simulink model "CCMMotor"  (R2022b) (continued)
== system CCMMotor/CCMMotor_Runnable_sys == (continued)
      settings: EnsureOutportIsVirtual=on
  AirFlapPosnFrntActForRowFirstForActrVentLe_AirFlapPosnFrntActForRowFirstForActrVentLe_write : Outport  Port=35
      settings: EnsureOutportIsVirtual=on
  AirFlapPosnFrntActForRowFirstForActrVentRi_AirFlapPosnFrntActForRowFirstForActrVentRi_write : Outport  Port=36
      settings: EnsureOutportIsVirtual=on
  AirFlapPosnFrntActForRowFirstForActrVentSideLe_AirFlapPosnFrntActForRowFirstForActrVentSideLe_write : Outport  Port=37
      settings: EnsureOutportIsVirtual=on
  AirFlapPosnFrntActForRowFirstForActrVentSideRi_AirFlapPosnFrntActForRowFirstForActrVentSideRi_write : Outport  Port=38
      settings: EnsureOutportIsVirtual=on
  AirFlapPosnFrntActForRowFirstForOsa_AirFlapPosnFrntActForRowFirstForOsa_write : Outport  Port=39
      settings: EnsureOutportIsVirtual=on
  AirFlapPosnFrntActForRowFirstForRecirc_AirFlapPosnFrntActForRowFirstForRecirc_write : Outport  Port=40
      settings: EnsureOutportIsVirtual=on
  AirFlapPosnFrntActForRowSecForActrTLe_AirFlapPosnFrntActForRowSecForActrTLe_write : Outport  Port=41
      settings: EnsureOutportIsVirtual=on
  AirFlapPosnFrntActForRowSecForActrTRi_AirFlapPosnFrntActForRowSecForActrTRi_write : Outport  Port=42
      settings: EnsureOutportIsVirtual=on
  CcmElecAirDirCrtlReqDrvrLePosX_CcmElecAirDirCrtlReqDrvrLePosX_write : Outport  Port=43
      settings: EnsureOutportIsVirtual=on
  CcmElecAirDirCrtlReqDrvrLePosY_CcmElecAirDirCrtlReqDrvrLePosY_write : Outport  Port=44
      settings: EnsureOutportIsVirtual=on
  CcmElecAirDirCrtlReqDrvrRiPosX_CcmElecAirDirCrtlReqDrvrRiPosX_write : Outport  Port=45
      settings: EnsureOutportIsVirtual=on
  CcmElecAirDirCrtlReqDrvrRiPosY_CcmElecAirDirCrtlReqDrvrRiPosY_write : Outport  Port=46
      settings: EnsureOutportIsVirtual=on
  CcmElecAirDirCrtlReqPassLePosX_CcmElecAirDirCrtlReqPassLePosX_write : Outport  Port=47
      settings: EnsureOutportIsVirtual=on
  CcmElecAirDirCrtlReqPassLePosY_CcmElecAirDirCrtlReqPassLePosY_write : Outport  Port=48
      settings: EnsureOutportIsVirtual=on
  CcmElecAirDirCrtlReqPassRiPosX_CcmElecAirDirCrtlReqPassRiPosX_write : Outport  Port=49
      settings: EnsureOutportIsVirtual=on
  CcmElecAirDirCrtlReqPassRiPosY_CcmElecAirDirCrtlReqPassRiPosY_write : Outport  Port=50
      settings: EnsureOutportIsVirtual=on
  FrntVGAerror_FrntVGAerror_write : Outport  Port=51
      settings: EnsureOutportIsVirtual=on
  RearVGAerror_RearVGAerror_write : Outport  Port=52
      settings: EnsureOutportIsVirtual=on
  VentnDrvrLeXStsRsp_VentnDrvrLeXStsRsp_write : Outport  Port=53
      settings: EnsureOutportIsVirtual=on
  VentnDrvrLeYStsRsp_VentnDrvrLeYStsRsp_write : Outport  Port=54
      settings: EnsureOutportIsVirtual=on
  VentnDrvrRiXStsRsp_VentnDrvrRiXStsRsp_write : Outport  Port=55
      settings: EnsureOutportIsVirtual=on
  VentnDrvrRiYStsRsp_VentnDrvrRiYStsRsp_write : Outport  Port=56
      settings: EnsureOutportIsVirtual=on
  VentnPassLeXStsRsp_VentnPassLeXStsRsp_write : Outport  Port=57
      settings: EnsureOutportIsVirtual=on
  VentnPassLeYStsRsp_VentnPassLeYStsRsp_write : Outport  Port=58
      settings: EnsureOutportIsVirtual=on
  VentnPassRiXStsRsp_VentnPassRiXStsRsp_write : Outport  Port=59
      settings: EnsureOutportIsVirtual=on
  VentnPassRiYStsRsp_VentnPassRiYStsRsp_write : Outport  Port=60
      settings: EnsureOutportIsVirtual=on
  VentnSecLeXStsRsp_VentnSecLeXStsRsp_write : Outport  Port=61
      settings: EnsureOutportIsVirtual=on
  VentnSecLeYStsRsp_VentnSecLeYStsRsp_write : Outport  Port=62
      settings: EnsureOutportIsVirtual=on
  VentnSecRiXStsRsp_VentnSecRiXStsRsp_write : Outport  Port=63
      settings: EnsureOutportIsVirtual=on
  VentnSecRiYStsRsp_VentnSecRiYStsRsp_write : Outport  Port=64
      settings: EnsureOutportIsVirtual=on
  wires:
  ActrFrntReqForRowSecModDistbnRi_ActrFrntReqForRowSecModDistbnRi_read/1 -> Terminator_1/1
  AirFlapPosnFrntReqForRowFirstForActrFlrLe_AirFlapPosnFrntReqForRowFirstForActrFlrLe_read/1 -> Terminator_2/1
  AirFlapPosnFrntReqForRowFirstForActrFlrRi_AirFlapPosnFrntReqForRowFirstForActrFlrRi_read/1 -> Terminator_3/1
  AirFlapPosnFrntReqForRowFirstForActrVentLe_AirFlapPosnFrntReqForRowFirstForActrVentLe_read/1 -> Terminator_4/1
  AirFlapPosnFrntReqForRowFirstForActrVentRi_AirFlapPosnFrntReqForRowFirstForActrVentRi_read/1 -> Terminator_5/1
  AirFlapPosnFrntReqForRowFirstForActrVentSideLe_AirFlapPosnFrntReqForRowFirstForActrVentSideLe_read/1 -> Terminator_6/1
  AirFlapPosnFrntReqForRowFirstForActrVentSideRi_AirFlapPosnFrntReqForRowFirstForActrVentSideRi_read/1 -> Terminator_7/1
  AirFlapPosnFrntReqForRowFirstForOsa_AirFlapPosnFrntReqForRowFirstForOsa_read/1 -> Terminator_8/1
  AirFlapPosnFrntReqForRowFirstForRecirc_AirFlapPosnFrntReqForRowFirstForRecirc_read/1 -> Terminator_9/1
  AirFlapPosnFrntReqForRowSecForActrTRi_AirFlapPosnFrntReqForRowSecForActrTRi_read/1 -> Terminator_10/1
  PwrMdAllMtrSlp_PwrMdAllMtrSlp_read/1 -> Terminator_11/1
  SysServCarCfgSts_SysServCarID_read/1 -> Terminator_12/1
  SysServCarCfgSts_SysServCCPAQSSts_read/1 -> Terminator_13/1
  SysServCarCfgSts_SysServCCPHEXSensorSts_read/1 -> Terminator_14/1
  SysServCarCfgSts_SysServCCPLin2AFUSts_read/1 -> Terminator_15/1
  SysServCarCfgSts_SysServCCPLin2AIUSts_read/1 -> Terminator_16/1
  SysServCarCfgSts_SysServCCPLin2CDSSts_read/1 -> Terminator_17/1
  SysServCarCfgSts_SysServCCPLin2PMSISts_read/1 -> Terminator_18/1
  SysServCarCfgSts_SysServCCPLin3VGASts_read/1 -> Terminator_19/1
  SysServCarCfgSts_SysServDUAirVentSnrSts_read/1 -> Terminator_20/1
  SysServCarCfgSts_SysServDUBlowerPwmSignSts_read/1 -> Terminator_21/1
  SysServCarCfgSts_SysServDUBlowerPwmSts_read/1 -> Terminator_22/1
  SysServCarCfgSts_SysServDUDCDefrostSts_read/1 -> Terminator_23/1
  SysServCarCfgSts_SysServDUDCModeSts_read/1 -> Terminator_24/1
  SysServCarCfgSts_SysServDUDCRecSts_read/1 -> Terminator_25/1
  SysServCarCfgSts_SysServDUDCSModeSts_read/1 -> Terminator_26/1
  SysServCarCfgSts_SysServDUDCSTLSts_read/1 -> Terminator_27/1
  SysServCarCfgSts_SysServDUDCTLSts_read/1 -> Terminator_28/1
  SysServCarCfgSts_SysServDUDCTRSts_read/1 -> Terminator_29/1
  SysServCarCfgSts_SysServDUFLFootSts_read/1 -> Terminator_30/1
  SysServCarCfgSts_SysServDUFLVentSts_read/1 -> Terminator_31/1
  SysServCarCfgSts_SysServDUFRFootSts_read/1 -> Terminator_32/1
  SysServCarCfgSts_SysServDUFRVentSts_read/1 -> Terminator_33/1
  SysServCarCfgSts_SysServDUIncarMotorSts_read/1 -> Terminator_34/1
  SysServCarCfgSts_SysServDULin1HBMFSts_read/1 -> Terminator_35/1
  SysServCarCfgSts_SysServDULin4HFAFSts_read/1 -> Terminator_36/1
  SysServCarCfgSts_SysServDUSFLFootSts_read/1 -> Terminator_37/1
  SysServCarCfgSts_SysServDUSFLVentSts_read/1 -> Terminator_38/1
  SysServCarCfgSts_SysServDUSFRFootSts_read/1 -> Terminator_39/1
  SysServCarCfgSts_SysServDUSFRVentSts_read/1 -> Terminator_40/1
  SysServCarCfgSts_SysServDUSnsrForFCmptmtTSts_read/1 -> Terminator_41/1
  SysServCarCfgSts_SysServDUSnsrForRair_read/1 -> Terminator_42/1
  SysServCarCfgSts_SysServDUSnsrForRCmptmtTSts_read/1 -> Terminator_43/1
  VehModMngtGlbSafe1_VehModMngtGlbSafe1_read/1 -> Terminator_44/1
  ReqForActrCal_ReqForActrCal_read/1 -> Terminator_45/1
  ActrFrntReqForRowFirstLeModDistbn_ActrFrntReqForRowFirstLeModDistbn_read/1 -> Terminator_46/1
  ActrFrntReqForRowFirstLeModDistbn_ActrFrntReqForRowFirstLeModDistbn_IsUpdated_read/1 -> Terminator_47/1
  ActrFrntReqForRowFirstModDistbn_ActrFrntReqForRowFirstModDistbn_read/1 -> Terminator_48/1
  ActrFrntReqForRowFirstModDistbn_ActrFrntReqForRowFirstModDistbn_IsUpdated_read/1 -> Terminator_49/1
  ActrFrntReqForRowSecModDistbnLe_ActrFrntReqForRowSecModDistbnLe_read/1 -> Terminator_50/1
  ActrFrntReqForRowSecModDistbnLe_ActrFrntReqForRowSecModDistbnLe_IsUpdated_read/1 -> Terminator_51/1
  ActrPosnFrnReqForOsaAndRecirc_ActrPosnFrnReqForOsaAndRecirc_read/1 -> Terminator_52/1
  ActrPosnFrnReqForOsaAndRecirc_ActrPosnFrnReqForOsaAndRecirc_IsUpdated_read/1 -> Terminator_53/1
  ActrReqForModDistbnRe_ActrReqForModDistbnRe_read/1 -> Terminator_54/1
  ActrReqForModDistbnRe_ActrReqForModDistbnRe_IsUpdated_read/1 -> Terminator_55/1
  AirFlapPosnFrntReqForRowFirstForActrDefrst_AirFlapPosnFrntReqForRowFirstForActrDefrst_read/1 -> Terminator_56/1
  AirFlapPosnFrntReqForRowFirstForActrDefrst_AirFlapPosnFrntReqForRowFirstForActrDefrst_IsUpdated_read/1 -> Terminator_57/1
  AirFlapPosnFrntReqForRowFirstForActrTLe_AirFlapPosnFrntReqForRowFirstForActrTLe_read/1 -> Terminator_58/1
  AirFlapPosnFrntReqForRowFirstForActrTLe_AirFlapPosnFrntReqForRowFirstForActrTLe_IsUpdated_read/1 -> Terminator_59/1
  AirFlapPosnFrntReqForRowFirstForActrTRi_AirFlapPosnFrntReqForRowFirstForActrTRi_read/1 -> Terminator_60/1
  AirFlapPosnFrntReqForRowFirstForActrTRi_AirFlapPosnFrntReqForRowFirstForActrTRi_IsUpdated_read/1 -> Terminator_61/1
  AirFlapPosnFrntReqForRowSecForActrTLe_AirFlapPosnFrntReqForRowSecForActrTLe_read/1 -> Terminator_62/1
  AirFlapPosnFrntReqForRowSecForActrTLe_AirFlapPosnFrntReqForRowSecForActrTLe_IsUpdated_read/1 -> Terminator_63/1
  CarCfgForClimaCtrl_CarCfgForAirClng_read/1 -> Terminator_64/1
  CarCfgForClimaCtrl_CarCfgForAirClnrAdv_read/1 -> Terminator_65/1
  CarCfgForClimaCtrl_CarCfgForAirFrag_read/1 -> Terminator_66/1
  CarCfgForClimaCtrl_CarCfgForAirMtrlSnsr_read/1 -> Terminator_67/1
  CarCfgForClimaCtrl_CarCfgForAirQly_read/1 -> Terminator_68/1
  CarCfgForClimaCtrl_CarCfgForAmntOfSeat_read/1 -> Terminator_69/1
  CarCfgForClimaCtrl_CarCfgForAutDemst_read/1 -> Terminator_70/1
  CarCfgForClimaCtrl_CarCfgForAuxWtrPmp_read/1 -> Terminator_71/1
  CarCfgForClimaCtrl_CarCfgForBRAND_read/1 -> Terminator_72/1
  CarCfgForClimaCtrl_CarCfgForBypVlvInHvac_read/1 -> Terminator_73/1
  CarCfgForClimaCtrl_CarCfgForClsVlvInHvac_read/1 -> Terminator_74/1
  CarCfgForClimaCtrl_CarCfgForCmprAcTyp_read/1 -> Terminator_75/1
  CarCfgForClimaCtrl_CarCfgForCmptmtClng_read/1 -> Terminator_76/1
  CarCfgForClimaCtrl_CarCfgForElectricLiftingModule_read/1 -> Terminator_77/1
  CarCfgForClimaCtrl_CarCfgForElecVentAdjmt_read/1 -> Terminator_78/1
  CarCfgForClimaCtrl_CarCfgForExtVentnClimaCtrl_read/1 -> Terminator_79/1
  CarCfgForClimaCtrl_CarCfgForFlcTyp_read/1 -> Terminator_80/1
  CarCfgForClimaCtrl_CarCfgForFoldCSD_read/1 -> Terminator_81/1
  CarCfgForClimaCtrl_CarCfgForFuTyp_read/1 -> Terminator_82/1
  CarCfgForClimaCtrl_CarCfgForGlassTyp_read/1 -> Terminator_83/1
  CarCfgForClimaCtrl_CarCfgForHeadRadar_read/1 -> Terminator_84/1
  CarCfgForClimaCtrl_CarCfgForHeatPmp_read/1 -> Terminator_85/1
  CarCfgForClimaCtrl_CarCfgForHeatrDurgDrvg_read/1 -> Terminator_86/1
  CarCfgForClimaCtrl_CarCfgForHeatrTyp_read/1 -> Terminator_87/1
  CarCfgForClimaCtrl_CarCfgForHvacFilTyp_read/1 -> Terminator_88/1
  CarCfgForClimaCtrl_CarCfgForHvacFlrSnsrForT_read/1 -> Terminator_89/1
  CarCfgForClimaCtrl_CarCfgForHvacHeatrSnsrT_read/1 -> Terminator_90/1
  CarCfgForClimaCtrl_CarCfgForHvacRe_read/1 -> Terminator_91/1
  CarCfgForClimaCtrl_CarCfgForHvacTyp_read/1 -> Terminator_92/1
  CarCfgForClimaCtrl_CarCfgForInteriorViewCamera_read/1 -> Terminator_93/1
  CarCfgForClimaCtrl_CarCfgForLifeDetection_read/1 -> Terminator_94/1
  CarCfgForClimaCtrl_CarCfgForLoVoltElecCfg_read/1 -> Terminator_95/1
  CarCfgForClimaCtrl_CarCfgForNavSysandIntnmap_read/1 -> Terminator_96/1
  CarCfgForClimaCtrl_CarCfgForNrOfDoor_read/1 -> Terminator_97/1
  CarCfgForClimaCtrl_CarCfgForPipeInFuTank_read/1 -> Terminator_98/1
  CarCfgForClimaCtrl_CarCfgForPowerSeats_read/1 -> Terminator_99/1
  CarCfgForClimaCtrl_CarCfgForPreClng_read/1 -> Terminator_100/1
  CarCfgForClimaCtrl_CarCfgForPrkgCDSNotAvl_read/1 -> Terminator_101/1
  CarCfgForClimaCtrl_CarCfgForPrkgClima_read/1 -> Terminator_102/1
  CarCfgForClimaCtrl_CarCfgForPrpsnTyp_read/1 -> Terminator_103/1
  CarCfgForClimaCtrl_CarCfgForPTCInFrontHVAC_read/1 -> Terminator_104/1
  CarCfgForClimaCtrl_CarCfgForRemEngStrtPreCdn_read/1 -> Terminator_105/1
  CarCfgForClimaCtrl_CarCfgForRoofHatch_read/1 -> Terminator_106/1
  CarCfgForClimaCtrl_CarCfgForSeatHeatgCal_read/1 -> Terminator_107/1
  CarCfgForClimaCtrl_CarCfgForSeatHeatgForRowFirst_read/1 -> Terminator_108/1
  CarCfgForClimaCtrl_CarCfgForSeatHeatgForRowSec_read/1 -> Terminator_109/1
  CarCfgForClimaCtrl_CarCfgForSeatVentnForRowFirst_read/1 -> Terminator_110/1
  CarCfgForClimaCtrl_CarCfgForSeatVentnForRowSec_read/1 -> Terminator_111/1
  CarCfgForClimaCtrl_CarCfgForSecRowVentnCenter_read/1 -> Terminator_112/1
  CarCfgForClimaCtrl_CarCfgForSecRowVentnSide_read/1 -> Terminator_113/1
  CarCfgForClimaCtrl_CarCfgForSmallFlowCyc_read/1 -> Terminator_114/1
  CarCfgForClimaCtrl_CarCfgForSmkSnsr_read/1 -> Terminator_115/1
  CarCfgForClimaCtrl_CarCfgForSnsrForCmptmtT_read/1 -> Terminator_116/1
  CarCfgForClimaCtrl_CarCfgForSnsrHumPrsnt_read/1 -> Terminator_117/1
  CarCfgForClimaCtrl_CarCfgForStartDevice_read/1 -> Terminator_118/1
  CarCfgForClimaCtrl_CarCfgForSteerWhlHeatg_read/1 -> Terminator_119/1
  CarCfgForClimaCtrl_CarCfgForSteerWhlPosn_read/1 -> Terminator_120/1
  CarCfgForClimaCtrl_CarCfgForSunSnsr_read/1 -> Terminator_121/1
  CarCfgForClimaCtrl_CarCfgForTelmNodTyp_read/1 -> Terminator_122/1
  CarCfgForClimaCtrl_CarCfgForTEMPEREDSTORAGEBOX_read/1 -> Terminator_123/1
  CarCfgForClimaCtrl_CarCfgForThermMngt_read/1 -> Terminator_124/1
  CarCfgForClimaCtrl_CarCfgForVehTyp_read/1 -> Terminator_125/1
  CarCfgForClimaCtrl_CarCfgForVentLiftMotorExiSts_read/1 -> Terminator_126/1
  CarCfgForClimaCtrl_CarCfgForVgaMotorExistSts_read/1 -> Terminator_127/1
  CarCfgForClimaCtrl_CarCfgForWadingAssistSystem_read/1 -> Terminator_128/1
  CarCfgForClimaCtrl_CarCfgForWindHeatd_read/1 -> Terminator_129/1
  CarCfgForClimaCtrl_CarCfgWindFollowHumanMov_read/1 -> Terminator_130/1
  SysServCarCfgSts_SysServCCPBGMNum_read/1 -> Terminator_131/1
  SysServCarCfgSts_SysServCCPLin2AQSSts_read/1 -> Terminator_132/1
  SysServCarCfgSts_SysServDUBlowerPwmSignTypeSts_read/1 -> Terminator_133/1
  SysServCarCfgSts_SysServDUDCFLMdeSts_read/1 -> Terminator_134/1
  SysServCarCfgSts_SysServDULin4HFAFStsH_read/1 -> Terminator_135/1
  SysServCarCfgSts_SysServDUReBlowerPwmSignSts_read/1 -> Terminator_136/1
  SysServCarCfgSts_SysServDUReBlowerPwmSts_read/1 -> Terminator_137/1
  SysServCarCfgSts_SysServFrntHEXSnsrPosn_read/1 -> Terminator_138/1
  SysServCarCfgSts_SysServLowSideRETXVSts_read/1 -> Terminator_139/1
  SysServCarCfgSts_SysServReHEXSnsrPosn_read/1 -> Terminator_140/1
  SysServCarCfgSts_SysServRHFASts_read/1 -> Terminator_141/1
  SysServCarCfgSts_SysServSnsrForFCmptmtTType_read/1 -> Terminator_142/1
  VentLiftModCaliReq_VentLiftModCaliReq_read/1 -> Terminator_143/1
  VentnDrvrLeXPsonReq_VentnDrvrLeXPsonReq_read/1 -> Terminator_144/1
  VentnDrvrLeXPsonReq_VentnDrvrLeXPsonReq_IsUpdated_read/1 -> Terminator_145/1
  VentnDrvrLeYPsonReq_VentnDrvrLeYPsonReq_read/1 -> Terminator_146/1
  VentnDrvrLeYPsonReq_VentnDrvrLeYPsonReq_IsUpdated_read/1 -> Terminator_147/1
  VentnDrvrRiXPsonReq_VentnDrvrRiXPsonReq_read/1 -> Terminator_148/1
  VentnDrvrRiXPsonReq_VentnDrvrRiXPsonReq_IsUpdated_read/1 -> Terminator_149/1
  VentnDrvrRiYPsonReq_VentnDrvrRiYPsonReq_read/1 -> Terminator_150/1
  VentnDrvrRiYPsonReq_VentnDrvrRiYPsonReq_IsUpdated_read/1 -> Terminator_151/1
  VentnLiftModPosnReq_VentnLiftModPosnReq_read/1 -> Terminator_152/1
  VentnLiftModPosnReq_VentnLiftModPosnReq_IsUpdated_read/1 -> Terminator_153/1
  VentnPassLeXPsonReq_VentnPassLeXPsonReq_read/1 -> Terminator_154/1
  VentnPassLeXPsonReq_VentnPassLeXPsonReq_IsUpdated_read/1 -> Terminator_155/1
  VentnPassLeYPsonReq_VentnPassLeYPsonReq_read/1 -> Terminator_156/1
  VentnPassLeYPsonReq_VentnPassLeYPsonReq_IsUpdated_read/1 -> Terminator_157/1
  VentnPassRiXPsonReq_VentnPassRiXPsonReq_read/1 -> Terminator_158/1
  VentnPassRiXPsonReq_VentnPassRiXPsonReq_IsUpdated_read/1 -> Terminator_159/1
  VentnPassRiYPsonReq_VentnPassRiYPsonReq_read/1 -> Terminator_160/1
  VentnPassRiYPsonReq_VentnPassRiYPsonReq_IsUpdated_read/1 -> Terminator_161/1
  VentnSecLeXPosnReq_VentnSecLeXPosnReq_read/1 -> Terminator_162/1
  VentnSecLeXPosnReq_VentnSecLeXPosnReq_IsUpdated_read/1 -> Terminator_163/1
  VentnSecLeYPosnReq_VentnSecLeYPosnReq_read/1 -> Terminator_164/1
  VentnSecLeYPosnReq_VentnSecLeYPosnReq_IsUpdated_read/1 -> Terminator_165/1
  VentnSecRiXPosnReq_VentnSecRiXPosnReq_read/1 -> Terminator_166/1
  VentnSecRiXPosnReq_VentnSecRiXPosnReq_IsUpdated_read/1 -> Terminator_167/1
  VentnSecRiYPosnReq_VentnSecRiYPosnReq_read/1 -> Terminator_168/1
  VentnSecRiYPosnReq_VentnSecRiYPosnReq_IsUpdated_read/1 -> Terminator_169/1
  WtrBafePsonReq_WtrBafePsonReq_read/1 -> Terminator_170/1
  BswLifeCycle_LifeCycle_read/1 -> Terminator_171/1
  DcMotorParaService_DcMotorAllowSleepSts/1 -> Terminator_172/1
  DcMotorParaService_DcMotorAllowSleepSts/2 -> Terminator_173/1
  Ground_1/1 -> DcMotorParaService_GetMotorActualPositon/1
  DcMotorParaService_GetMotorActualPositon/1 -> Terminator_174/1
  DcMotorParaService_GetMotorActualPositon/2 -> Terminator_175/1
  DcMotorParaService_GetMotorCailLearnSts/1 -> Terminator_176/1
  DcMotorParaService_GetMotorCailLearnSts/2 -> Terminator_177/1
  Ground_2/1 -> DcMotorParaService_GetMotorCalibrationStatus/1
  DcMotorParaService_GetMotorCalibrationStatus/1 -> Terminator_178/1
  DcMotorParaService_GetMotorCalibrationStatus/2 -> Terminator_179/1
  Ground_3/1 -> DcMotorParaService_GetMotorDidWorkingStatus/1
  DcMotorParaService_GetMotorDidWorkingStatus/1 -> Terminator_180/1
  DcMotorParaService_GetMotorDidWorkingStatus/2 -> Terminator_181/1
  Ground_4/1 -> DcMotorParaService_GetMotorEndPosition/1
  DcMotorParaService_GetMotorEndPosition/1 -> Terminator_182/1
  DcMotorParaService_GetMotorEndPosition/2 -> Terminator_183/1
  Ground_5/1 -> DcMotorParaService_GetMotorRequestPercent/1
  DcMotorParaService_GetMotorRequestPercent/1 -> Terminator_184/1
  DcMotorParaService_GetMotorRequestPercent/2 -> Terminator_185/1
  DcMotorParaService_GetMotorRoutineCailSts/1 -> Terminator_186/1
  DcMotorParaService_GetMotorRoutineCailSts/2 -> Terminator_187/1
  Ground_6/1 -> DcMotorParaService_GetMotorRunTime/1
  DcMotorParaService_GetMotorRunTime/1 -> Terminator_188/1
  DcMotorParaService_GetMotorRunTime/2 -> Terminator_189/1
  Ground_7/1 -> DcMotorParaService_GetMotorWorkingStatus/1
  DcMotorParaService_GetMotorWorkingStatus/1 -> Terminator_190/1
  DcMotorParaService_GetMotorWorkingStatus/2 -> Terminator_191/1
  Ground_8/1 -> DcMotorParaService_GetMotorZeroPosition/1
  DcMotorParaService_GetMotorZeroPosition/1 -> Terminator_192/1
  DcMotorParaService_GetMotorZeroPosition/2 -> Terminator_193/1
  DcMotorParaService_GetRamAirErrorStatus/1 -> Terminator_194/1
  DcMotorParaService_GetRamAirErrorStatus/2 -> Terminator_195/1
  Ground_9/1 -> DcMotorParaService_SetMotorEndPosition/1
  Ground_10/1 -> DcMotorParaService_SetMotorEndPosition/2
  DcMotorParaService_SetMotorEndPosition/1 -> Terminator_196/1
  Ground_11/1 -> DcMotorParaService_SetMotorRequestPercent/1
  Ground_12/1 -> DcMotorParaService_SetMotorRequestPercent/2
  DcMotorParaService_SetMotorRequestPercent/1 -> Terminator_197/1
  DcMotorParaService_SetMotorRoutineCail/1 -> Terminator_198/1
  Ground_13/1 -> DcMotorParaService_SetMotorRoutineCailSts/1
  DcMotorParaService_SetMotorRoutineCailSts/1 -> Terminator_199/1
  Ground_14/1 -> DcMotorParaService_SetMotorRunTime/1
  Ground_15/1 -> DcMotorParaService_SetMotorRunTime/2
  DcMotorParaService_SetMotorRunTime/1 -> Terminator_200/1
  Ground_16/1 -> DcMotorParaService_SetMotorZeroPosition/1
  Ground_17/1 -> DcMotorParaService_SetMotorZeroPosition/2
  DcMotorParaService_SetMotorZeroPosition/1 -> Terminator_201/1
  DcMtrCaliServive_SetAllCalibrate/1 -> Terminator_202/1
  Ground_18/1 -> DcMtrCaliServive_SetMotorCalibrate/1
  DcMtrCaliServive_SetMotorCalibrate/1 -> Terminator_203/1
  HfaCaliService_SetAllCalibrate/1 -> Terminator_204/1
  HfaCaliService_SetAllParaInit/1 -> Terminator_205/1
  Ground_19/1 -> HfaCaliService_SetMotorCalibrate/1
  HfaCaliService_SetMotorCalibrate/1 -> Terminator_206/1
  Ground_20/1 -> HfaCaliService_SetMotorParaInit/1
  HfaCaliService_SetMotorParaInit/1 -> Terminator_207/1
  HfaParaService_GetAllMotorCaliStatus/1 -> Terminator_208/1
  HfaParaService_GetAllMotorCaliStatus/2 -> Terminator_209/1
  HfaParaService_GetCailRoutineStatus/1 -> Terminator_210/1
  HfaParaService_GetCailRoutineStatus/2 -> Terminator_211/1
  HfaParaService_GetFreshRecErrorStatus/1 -> Terminator_212/1
  HfaParaService_GetFreshRecErrorStatus/2 -> Terminator_213/1
  HfaParaService_GetMotorAllowSleepSts/1 -> Terminator_214/1
  HfaParaService_GetMotorAllowSleepSts/2 -> Terminator_215/1
  Ground_21/1 -> HfaParaService_GetMotorCaliStatus/1
  HfaParaService_GetMotorCaliStatus/1 -> Terminator_216/1
  HfaParaService_GetMotorCaliStatus/2 -> Terminator_217/1
  Ground_22/1 -> HfaParaService_GetMotorCurrentPosition/1
  HfaParaService_GetMotorCurrentPosition/1 -> Terminator_218/1
  HfaParaService_GetMotorCurrentPosition/2 -> Terminator_219/1
  Ground_23/1 -> HfaParaService_GetMotorDidWorkingStatus/1
  HfaParaService_GetMotorDidWorkingStatus/1 -> Terminator_220/1
  HfaParaService_GetMotorDidWorkingStatus/2 -> Terminator_221/1
  Ground_24/1 -> HfaParaService_GetMotorMoveCnt/1
  HfaParaService_GetMotorMoveCnt/1 -> Terminator_222/1
  HfaParaService_GetMotorMoveCnt/2 -> Terminator_223/1
  Ground_25/1 -> HfaParaService_GetMotorReqPosition/1
  HfaParaService_GetMotorReqPosition/1 -> Terminator_224/1
  HfaParaService_GetMotorReqPosition/2 -> Terminator_225/1
  Ground_26/1 -> HfaParaService_GetMotorStepRange/1
  HfaParaService_GetMotorStepRange/1 -> Terminator_226/1
  HfaParaService_GetMotorStepRange/2 -> Terminator_227/1
  Ground_27/1 -> HfaParaService_GetMotorWorkingStatus/1
  HfaParaService_GetMotorWorkingStatus/1 -> Terminator_228/1
  HfaParaService_GetMotorWorkingStatus/2 -> Terminator_229/1
  HfaParaService_GetRamAirErrorStatus/1 -> Terminator_230/1
  HfaParaService_GetRamAirErrorStatus/2 -> Terminator_231/1
  Ground_28/1 -> HfaParaService_SetCailRoutineStatus/1
  HfaParaService_SetCailRoutineStatus/1 -> Terminator_232/1
  Ground_29/1 -> HfaParaService_SetMotorMoveCnt/1
  Ground_30/1 -> HfaParaService_SetMotorMoveCnt/2
  HfaParaService_SetMotorMoveCnt/1 -> Terminator_233/1
  Ground_31/1 -> HfaParaService_SetMotorRequestPosition/1
  Ground_32/1 -> HfaParaService_SetMotorRequestPosition/2
  HfaParaService_SetMotorRequestPosition/1 -> Terminator_234/1
  Ground_33/1 -> HfaParaService_SetMotorStepRange/1
  Ground_34/1 -> HfaParaService_SetMotorStepRange/2
  HfaParaService_SetMotorStepRange/1 -> Terminator_235/1
  VgaCaliServive_SetAllCalibrate/1 -> Terminator_236/1
  VgaCaliServive_SetAllParaInit/1 -> Terminator_237/1
  Ground_35/1 -> VgaCaliServive_SetMotorCalibrate/1
  VgaCaliServive_SetMotorCalibrate/1 -> Terminator_238/1
  Ground_36/1 -> VgaCaliServive_SetMotorCalibrateNew/1
  Ground_37/1 -> VgaCaliServive_SetMotorCalibrateNew/2
  VgaCaliServive_SetMotorCalibrateNew/1 -> Terminator_239/1
  Ground_38/1 -> VgaCaliServive_SetMotorParaInit/1
  VgaCaliServive_SetMotorParaInit/1 -> Terminator_240/1
  VgaParaService_GetAllMotorCaliStatus/1 -> Terminator_241/1
  VgaParaService_GetAllMotorCaliStatus/2 -> Terminator_242/1
  VgaParaService_GetCailRoutineStatus/1 -> Terminator_243/1
  VgaParaService_GetCailRoutineStatus/2 -> Terminator_244/1
  VgaParaService_GetFreshRecErrorStatus/1 -> Terminator_245/1
  VgaParaService_GetFreshRecErrorStatus/2 -> Terminator_246/1
  VgaParaService_GetFrntVgaErrorStatus/1 -> Terminator_247/1
  VgaParaService_GetFrntVgaErrorStatus/2 -> Terminator_248/1
  VgaParaService_GetMotorAllowSleepSts/1 -> Terminator_249/1
  VgaParaService_GetMotorAllowSleepSts/2 -> Terminator_250/1
  Ground_39/1 -> VgaParaService_GetMotorCaliStatus/1
  VgaParaService_GetMotorCaliStatus/1 -> Terminator_251/1
  VgaParaService_GetMotorCaliStatus/2 -> Terminator_252/1
  Ground_40/1 -> VgaParaService_GetMotorCurrentPosition/1
  VgaParaService_GetMotorCurrentPosition/1 -> Terminator_253/1
  VgaParaService_GetMotorCurrentPosition/2 -> Terminator_254/1
  Ground_41/1 -> VgaParaService_GetMotorCurrentPositionNew/1
  VgaParaService_GetMotorCurrentPositionNew/1 -> Terminator_255/1
  VgaParaService_GetMotorCurrentPositionNew/2 -> Terminator_256/1
  Ground_42/1 -> VgaParaService_GetMotorDidWorkingStatus/1
  VgaParaService_GetMotorDidWorkingStatus/1 -> Terminator_257/1
  VgaParaService_GetMotorDidWorkingStatus/2 -> Terminator_258/1
  Ground_43/1 -> VgaParaService_GetMotorMoveCnt/1
  VgaParaService_GetMotorMoveCnt/1 -> Terminator_259/1
  VgaParaService_GetMotorMoveCnt/2 -> Terminator_260/1
  Ground_44/1 -> VgaParaService_GetMotorReqPosition/1
  VgaParaService_GetMotorReqPosition/1 -> Terminator_261/1
  VgaParaService_GetMotorReqPosition/2 -> Terminator_262/1
  Ground_45/1 -> VgaParaService_GetMotorStepRange/1
  VgaParaService_GetMotorStepRange/1 -> Terminator_263/1
  VgaParaService_GetMotorStepRange/2 -> Terminator_264/1
  Ground_46/1 -> VgaParaService_GetMotorWorkingStatus/1
  VgaParaService_GetMotorWorkingStatus/1 -> Terminator_265/1
  VgaParaService_GetMotorWorkingStatus/2 -> Terminator_266/1
  VgaParaService_GetRearVgaErrorStatus/1 -> Terminator_267/1
  VgaParaService_GetRearVgaErrorStatus/2 -> Terminator_268/1
  Ground_47/1 -> VgaParaService_SetCailRoutineStatus/1
  VgaParaService_SetCailRoutineStatus/1 -> Terminator_269/1
  Ground_48/1 -> VgaParaService_SetMotorMoveCnt/1
  Ground_49/1 -> VgaParaService_SetMotorMoveCnt/2
  VgaParaService_SetMotorMoveCnt/1 -> Terminator_270/1
  Ground_50/1 -> VgaParaService_SetMotorRequestPosition/1
  Ground_51/1 -> VgaParaService_SetMotorRequestPosition/2
  VgaParaService_SetMotorRequestPosition/1 -> Terminator_271/1
  Ground_52/1 -> VgaParaService_SetMotorStepRange/1
  Ground_53/1 -> VgaParaService_SetMotorStepRange/2
  VgaParaService_SetMotorStepRange/1 -> Terminator_272/1
  Ground_54/1 -> ActrPosnActForModDistbnRe_ActrPosnActForModDistbnRe_write/1
  Ground_55/1 -> ActrPosnFrntActForRowFirstModDistbnLe_ActrPosnFrntActForRowFirstModDistbnLe_write/1
  Ground_56/1 -> AirFlapFrntStsForRowFirstModDistbnLe_AirFlapFrntStsForRowFirstModDistbnLe_write/1
  Ground_57/1 -> AirFlapStsForActModDistbnRe_AirFlapStsForActModDistbnRe_write/1
  Ground_58/1 -> DriverVolThresholdLimitOfMotor_DriverVolThresholdLimitOfMotor_write/1
  Ground_59/1 -> HvacOsaAndRecActrQfForHp_HvacOsaAndRecActrQfForHp_write/1
  Ground_60/1 -> VentnLiftModPosnRsp_VentnLiftModPosnRsp_write/1
  Ground_61/1 -> VentnLiftModStsRsp_VentnLiftModStsRsp_write/1
  Ground_62/1 -> ActrFrntStsForRowFirstModDistbn_ActrFrntStsForRowFirstModDistbn_write/1
  Ground_63/1 -> ActrFrntStsForRowSecModDistbnLe_ActrFrntStsForRowSecModDistbnLe_write/1
  Ground_64/1 -> ActrFrntStsForRowSecModDistbnRi_ActrFrntStsForRowSecModDistbnRi_write/1
  Ground_65/1 -> ActrPosnFrntActForOsaAndRecirc_ActrPosnFrntActForOsaAndRecirc_write/1
  Ground_66/1 -> ActrPosnFrntActForRowFirstModDistbn_ActrPosnFrntActForRowFirstModDistbn_write/1
  Ground_67/1 -> ActrPosnFrntActForRowSecModDistbnLe_ActrPosnFrntActForRowSecModDistbnLe_write/1
  Ground_68/1 -> ActrPosnFrntActForRowSecModDistbnRi_ActrPosnFrntActForRowSecModDistbnRi_write/1
  Ground_69/1 -> AirFlapFrntStsForRowFirstForActrDefrst_AirFlapFrntStsForRowFirstForActrDefrst_write/1
  Ground_70/1 -> AirFlapFrntStsForRowFirstForActrFlrLe_AirFlapFrntStsForRowFirstForActrFlrLe_write/1
  Ground_71/1 -> AirFlapFrntStsForRowFirstForActrFlrRi_AirFlapFrntStsForRowFirstForActrFlrRi_write/1
  Ground_72/1 -> AirFlapFrntStsForRowFirstForActrTLe_AirFlapFrntStsForRowFirstForActrTLe_write/1
  Ground_73/1 -> AirFlapFrntStsForRowFirstForActrTRi_AirFlapFrntStsForRowFirstForActrTRi_write/1
  Ground_74/1 -> AirFlapFrntStsForRowFirstForActrVentLe_AirFlapFrntStsForRowFirstForActrVentLe_write/1
  Ground_75/1 -> AirFlapFrntStsForRowFirstForActrVentRi_AirFlapFrntStsForRowFirstForActrVentRi_write/1
  Ground_76/1 -> AirFlapFrntStsForRowFirstForActrVentSideLe_AirFlapFrntStsForRowFirstForActrVentSideLe_write/1
  Ground_77/1 -> AirFlapFrntStsForRowFirstForActrVentSideRi_AirFlapFrntStsForRowFirstForActrVentSideRi_write/1
  Ground_78/1 -> AirFlapFrntStsForRowFirstForOsa_AirFlapFrntStsForRowFirstForOsa_write/1
  Ground_79/1 -> AirFlapFrntStsForRowFirstForOsaAndRecirc_AirFlapFrntStsForRowFirstForOsaAndRecirc_write/1
  Ground_80/1 -> AirFlapFrntStsForRowFirstForRecirc_AirFlapFrntStsForRowFirstForRecirc_write/1
  Ground_81/1 -> AirFlapFrntStsForRowSecForActrTLe_AirFlapFrntStsForRowSecForActrTLe_write/1
  Ground_82/1 -> AirFlapFrntStsForRowSecForActrTRi_AirFlapFrntStsForRowSecForActrTRi_write/1
  Ground_83/1 -> AirFlapPosnFrntActForRowFirstForActrDefrst_AirFlapPosnFrntActForRowFirstForActrDefrst_write/1
  Ground_84/1 -> AirFlapPosnFrntActForRowFirstForActrFlrLe_AirFlapPosnFrntActForRowFirstForActrFlrLe_write/1
  Ground_85/1 -> AirFlapPosnFrntActForRowFirstForActrFlrRi_AirFlapPosnFrntActForRowFirstForActrFlrRi_write/1
  Ground_86/1 -> AirFlapPosnFrntActForRowFirstForActrTLe_AirFlapPosnFrntActForRowFirstForActrTLe_write/1
  Ground_87/1 -> AirFlapPosnFrntActForRowFirstForActrTRi_AirFlapPosnFrntActForRowFirstForActrTRi_write/1
  Ground_88/1 -> AirFlapPosnFrntActForRowFirstForActrVentLe_AirFlapPosnFrntActForRowFirstForActrVentLe_write/1
  Ground_89/1 -> AirFlapPosnFrntActForRowFirstForActrVentRi_AirFlapPosnFrntActForRowFirstForActrVentRi_write/1
  Ground_90/1 -> AirFlapPosnFrntActForRowFirstForActrVentSideLe_AirFlapPosnFrntActForRowFirstForActrVentSideLe_write/1
  Ground_91/1 -> AirFlapPosnFrntActForRowFirstForActrVentSideRi_AirFlapPosnFrntActForRowFirstForActrVentSideRi_write/1
  Ground_92/1 -> AirFlapPosnFrntActForRowFirstForOsa_AirFlapPosnFrntActForRowFirstForOsa_write/1
  Ground_93/1 -> AirFlapPosnFrntActForRowFirstForRecirc_AirFlapPosnFrntActForRowFirstForRecirc_write/1
  Ground_94/1 -> AirFlapPosnFrntActForRowSecForActrTLe_AirFlapPosnFrntActForRowSecForActrTLe_write/1
  Ground_95/1 -> AirFlapPosnFrntActForRowSecForActrTRi_AirFlapPosnFrntActForRowSecForActrTRi_write/1
  Ground_96/1 -> CcmElecAirDirCrtlReqDrvrLePosX_CcmElecAirDirCrtlReqDrvrLePosX_write/1
  Ground_97/1 -> CcmElecAirDirCrtlReqDrvrLePosY_CcmElecAirDirCrtlReqDrvrLePosY_write/1
  Ground_98/1 -> CcmElecAirDirCrtlReqDrvrRiPosX_CcmElecAirDirCrtlReqDrvrRiPosX_write/1
  Ground_99/1 -> CcmElecAirDirCrtlReqDrvrRiPosY_CcmElecAirDirCrtlReqDrvrRiPosY_write/1
  Ground_100/1 -> CcmElecAirDirCrtlReqPassLePosX_CcmElecAirDirCrtlReqPassLePosX_write/1
  Ground_101/1 -> CcmElecAirDirCrtlReqPassLePosY_CcmElecAirDirCrtlReqPassLePosY_write/1
  Ground_102/1 -> CcmElecAirDirCrtlReqPassRiPosX_CcmElecAirDirCrtlReqPassRiPosX_write/1
  Ground_103/1 -> CcmElecAirDirCrtlReqPassRiPosY_CcmElecAirDirCrtlReqPassRiPosY_write/1
  Ground_104/1 -> FrntVGAerror_FrntVGAerror_write/1
  Ground_105/1 -> RearVGAerror_RearVGAerror_write/1
  Ground_106/1 -> VentnDrvrLeXStsRsp_VentnDrvrLeXStsRsp_write/1
  Ground_107/1 -> VentnDrvrLeYStsRsp_VentnDrvrLeYStsRsp_write/1
  Ground_108/1 -> VentnDrvrRiXStsRsp_VentnDrvrRiXStsRsp_write/1
  Ground_109/1 -> VentnDrvrRiYStsRsp_VentnDrvrRiYStsRsp_write/1
  Ground_110/1 -> VentnPassLeXStsRsp_VentnPassLeXStsRsp_write/1
  Ground_111/1 -> VentnPassLeYStsRsp_VentnPassLeYStsRsp_write/1
  Ground_112/1 -> VentnPassRiXStsRsp_VentnPassRiXStsRsp_write/1
  Ground_113/1 -> VentnPassRiYStsRsp_VentnPassRiYStsRsp_write/1
  Ground_114/1 -> VentnSecLeXStsRsp_VentnSecLeXStsRsp_write/1
  Ground_115/1 -> VentnSecLeYStsRsp_VentnSecLeYStsRsp_write/1
  Ground_116/1 -> VentnSecRiXStsRsp_VentnSecRiXStsRsp_write/1
  Ground_117/1 -> VentnSecRiYStsRsp_VentnSecRiYStsRsp_write/1
